simulink model "Demo_TrafficFlow_cs"  (Simulink 7.6)
settings: Solver=ode5  SolverMode=SingleTasking  StartTime=0.0  StopTime=60  FixedStep=1/20  RelTol=1e-3
PreLoadFcn:
  | check_NUTS_defined
  | try
  |     evalin('base', 'NUTS_defined;');
  | catch
  |     warningMsg = sprintf('PreScan Warning: \nUsing this model requires that MATLAB is configured for PreScan. The model may not display correctly.\n\n ==> Please open MATLAB with the dedicated buttons provided with PreScan');
  |     disp(warningMsg)
  |     warndlg(warningMsg,'WindowStyle','Modal');
  | end
  | 
  | model_closed = 0;
  | assignin('base',['model_closed_' bdroot],false);
InitFcn:
  | PreScanExperimentInitFcn(bdroot)
StopFcn:
  | PreScanExperimentStopFcn(bdroot)
undefined symbols (not defined in the file or in its folder): Advised_Speed_L1, Advised_Speed_L2, Advised_Speed_L3, DiscIntNeverNeededParam, spare_10, spare_5, spare_6, spare_7, spare_8, spare_9, State_L2, Throughput_L1, Throughput_L2, Throughput_L3, Velocity_L1, Velocity_L2, Velocity_L3
libraries: simulink x58, PreScanSimulinkLibrary x40, simulink_extras x32, PreScanUsefulBlocks x22, simulink_need_slupdate x3
(part 17 of 20)

== system Demo_TrafficFlow_cs/Toyota_Yaris_1/Simple_Longitudinal_ACC/LONGITUDINAL ACC & Dynamics ==
  note: DYNAMICS
  note: CONTROL
  note: CASE: DETECTION
      | vehicle in front detected
      | * postion control
      | * velocity control
  note: CASE: NO DETECTION
      | no vehicle in front detected
      | * velocity control
  Detected Range [m] : Inport
  Detected Velocity Vehicle [m/s] : Inport  Port=2
  Own Velocity Intention [km/h] : Inport  Port=3
  Safety Distance [m] : Inport  Port=4
  Gain1 : Gain  Gain=0.35
  Gain2 : Gain  Gain=1/3.6
  Rate Limiter2 : RateLimiter  RisingSlewLimit=3  FallingSlewLimit=-3  SampleTimeMode=inherited
  Sum : Sum  Inputs=-+|
  Sum2 : Sum  Inputs=++|
  Switch : Switch  Threshold=0.0001
  only forward driving : Saturate  UpperLimit=inf  LowerLimit=0
  Velocity [m/s] : Outport
  wires:
  Rate Limiter2/1 -> only forward driving/1
  only forward driving/1 -> Velocity [m/s]/1
  Sum/1 -> Gain1/1  "Control Error"
  Switch/1 -> Rate Limiter2/1
  Detected Range [m]/1 -> Switch/2, Sum/2
  Own Velocity Intention [km/h]/1 -> Gain2/1
  Gain2/1 -> Switch/3
  Sum2/1 -> Switch/1
  Detected Velocity Vehicle [m/s]/1 -> Sum2/2
  Safety Distance [m]/1 -> Sum/1
  Gain1/1 -> Sum2/1

== system Demo_TrafficFlow_cs/Toyota_Yaris_1/Trajectories/END OF TRACK REACHED & GO TO BUFFER ==
  Elapsed_Track [m] : Inport
  Length_of_Track [m] : Inport  Port=2
  Product1 : Product  RndMeth=Zero
  Relational Operator : RelationalOperator  OutDataTypeStr=boolean
  offset for displacement to  buffer of traffic LANE 3 : Constant  Tag=TrajSubSelector  Value=[0 27 0 0 0 0 0 0 0]
  Buffer_offset [m] : Outport
  End_of_Track_Reached [-] : Outport  Port=2
  wires:
  Length_of_Track [m]/1 -> Relational Operator/2
  Product1/1 -> Buffer_offset [m]/1
  Elapsed_Track [m]/1 -> Relational Operator/1
  Relational Operator/1 -> End_of_Track_Reached [-]/1, Product1/2
  offset for displacement to  buffer of traffic LANE 3/1 -> Product1/1

== system Demo_TrafficFlow_cs/Toyota_Yaris_1/Trajectories/ElapsedTrack1 ==
  Offset : Inport
  s : Inport  Port=2
  Mux : Mux  Inputs=3
  Mux1 : Mux  Inputs=3
  MuxState : Subsystem
  OffsetCor : Subsystem
  pitchAngle : Lookup  InputValues=s  Table=pitch  LookUpMeth=Interpolation-Use End Values
  rollAngle : Lookup  InputValues=s  Table=roll  LookUpMeth=Interpolation-Use End Values
  xpos : Lookup  InputValues=s  Table=x  LookUpMeth=Interpolation-Use End Values
  yawAngle : Lookup  InputValues=s  Table=yaw  LookUpMeth=Interpolation-Use End Values
  ypos : Lookup  InputValues=s  Table=y  LookUpMeth=Interpolation-Use End Values
  zpos : Lookup  InputValues=s  Table=z  LookUpMeth=Interpolation-Use End Values
  state : Outport
  wires:
  zpos/1 -> OffsetCor/3(z pos)
  rollAngle/1 -> OffsetCor/4(R angle)
  pitchAngle/1 -> OffsetCor/5(P angle)
  yawAngle/1 -> OffsetCor/6(Y angle)
  OffsetCor/6(Y angle_cor) -> Mux1/3
  OffsetCor/5(P angle_cor) -> Mux1/2
  OffsetCor/4(R angle_cor) -> Mux1/1
  OffsetCor/3(z pos_cor) -> Mux/3
  MuxState/1(State) -> state/1
  Mux/1 -> MuxState/1(xyzPos_[m])
  s/1 -> pitchAngle/1, yawAngle/1, rollAngle/1, zpos/1, ypos/1, xpos/1
  Offset/1 -> OffsetCor/7(Offset)
  xpos/1 -> OffsetCor/1(x pos)
  ypos/1 -> OffsetCor/2(y pos)
  OffsetCor/1(x pos_cor) -> Mux/1
  OffsetCor/2(y pos_cor) -> Mux/2
  Mux1/1 -> MuxState/2(RPYAngle_[rad])

== system Demo_TrafficFlow_cs/Toyota_Yaris_1/Trajectories/LANE CHANGE LEFT (cummulative y position;  multiple shift to right are possible) ==
  reset cum value : Inport
  velocity [m/s] : Inport  Port=2
  on/off [-] : Inport  Port=3
  Cumulative Lane Change : Subsystem
  Single LaneChange_Left : Subsystem
  Trigger : Subsystem  TreatAsAtomicUnit=on
  cum LC : Outport
  wires:
  Cumulative Lane Change/1(cum LC value) -> cum LC/1
  Single LaneChange_Left/2(single LC value) -> Cumulative Lane Change/3(single LC value)  "single LC value"
  reset cum value/1 -> Cumulative Lane Change/1(reset cum value)  "reset_signal"
  velocity [m/s]/1 -> Single LaneChange_Left/2(velocity [m/s])
  Trigger/1(Out1) -> Single LaneChange_Left/Enable
  Single LaneChange_Left/1(end LC reached) -> Cumulative Lane Change/2(end LC reached)  "end_reached"
  on/off [-]/1 -> Trigger/Trigger, Single LaneChange_Left/1(on/off [-])  "lane_change_signal"

== system Demo_TrafficFlow_cs/Toyota_Yaris_1/Trajectories/PathFollowerSva_7 ==
  From Workspace : FromWorkspace  VariableName=trajectory  SampleTime=TimeStep  OutputAfterFinalValue=Holding final value
  From Workspace1 : FromWorkspace  VariableName=trajectory  SampleTime=TimeStep  OutputAfterFinalValue=Setting to zero
  Mux : Mux  Inputs=2
  Selector1 : Selector  InputPortWidth=24  IndexOptions=Index vector (dialog)  Indices=[7:18]  OutputSizes=1
  Selector9 : Selector  InputPortWidth=24  IndexOptions=Index vector (dialog)  Indices=[1:6]  OutputSizes=1
  trajectory : Outport
  wires:
  Selector1/1 -> Mux/2
  Selector9/1 -> Mux/1
  Mux/1 -> trajectory/1
  From Workspace1/1 -> Selector1/1
  From Workspace/1 -> Selector9/1

== system Demo_TrafficFlow_cs/Toyota_Yaris_1/Trajectories/RESTART TRAJECT ==
  End_of_Track_Reached [-] : Inport
  ID_start_again [-] : Inport  Port=2
  objectID [-] : Inport  Port=3
  Logical Operator : Logic  AllPortsSameDT=off  OutDataTypeStr=boolean
  Memory : Memory
  Relational Operator1 : RelationalOperator  Operator===  OutDataTypeStr=boolean
  reset [-] : Outport
  Start_Traject [-] : Outport  Port=2
  wires:
  ID_start_again [-]/1 -> Relational Operator1/1
  Relational Operator1/1 -> reset [-]/1, Logical Operator/2
  Memory/1 -> Start_Traject [-]/1
  End_of_Track_Reached [-]/1 -> Logical Operator/1
  Logical Operator/1 -> Memory/1
  objectID [-]/1 -> Relational Operator1/2

== system Demo_TrafficFlow_cs/Toyota_Yaris_1/Trajectories/Random  LC generator ==
  Random Number : RandomNumber  Mean=0.5  Variance=0.1  SampleTime=0
  Rounding Function : Rounding  Operator=round
  0/1 : Outport
  wires:
  Rounding Function/1 -> 0/1/1
  Random Number/1 -> Rounding Function/1

== system Demo_TrafficFlow_cs/Toyota_Yaris_1/Trajectories/Traj2State1 ==
  Traj Org : Inport
  Offset : Inport  Port=2
  Add2 : Sum
  ApplyRotation : PreScanSimulinkLibrary/ApplyRotation  SystemSampleTime=-1
  ApplyRotation1 : PreScanSimulinkLibrary/ApplyRotation  SystemSampleTime=-1
  ApplyRotation2 : PreScanSimulinkLibrary/ApplyRotation  SystemSampleTime=-1
  Constant : Constant  Value=zeros(12,1)
  Mux : Mux  Inputs=6
  Mux1 : Mux  Inputs=2
  Mux{2,4..9} (7 alike) : Mux  Inputs=3
  Mux3 : Mux  Inputs=2
  Selector : Selector  InputPortWidth=6  IndexOptions=Index vector (dialog)  Indices=[4 5 6]  OutputSizes=1
  Selector1 : Selector  InputPortWidth=21  IndexOptions=Index vector (dialog)  Indices=[5]  OutputSizes=1
  Selector10 : Selector  InputPortWidth=21  IndexOptions=Index vector (dialog)  Indices=[2]  OutputSizes=1
  Selector11 : Selector  InputPortWidth=21  IndexOptions=Index vector (dialog)  Indices=[3]  OutputSizes=1
  Selector12 : Selector  InputPortWidth=21  IndexOptions=Index vector (dialog)  Indices=[1]  OutputSizes=1
  Selector13 : Selector  InputPortWidth=21  IndexOptions=Index vector (dialog)  Indices=[8]  OutputSizes=1
  Selector14 : Selector  InputPortWidth=21  IndexOptions=Index vector (dialog)  Indices=[9]  OutputSizes=1
  Selector15 : Selector  InputPortWidth=21  IndexOptions=Index vector (dialog)  Indices=[7]  OutputSizes=1
  Selector16 : Selector  InputPortWidth=21  IndexOptions=Index vector (dialog)  Indices=[14]  OutputSizes=1
  Selector17 : Selector  InputPortWidth=21  IndexOptions=Index vector (dialog)  Indices=[15]  OutputSizes=1
  Selector18 : Selector  InputPortWidth=21  IndexOptions=Index vector (dialog)  Indices=[13]  OutputSizes=1
  Selector19 : Selector  InputPortWidth=21  IndexOptions=Index vector (dialog)  Indices=[20]  OutputSizes=1
  Selector2 : Selector  InputPortWidth=21  IndexOptions=Index vector (dialog)  Indices=[6]  OutputSizes=1
  Selector20 : Selector  InputPortWidth=21  IndexOptions=Index vector (dialog)  Indices=[21]  OutputSizes=1
  Selector21 : Selector  InputPortWidth=21  IndexOptions=Index vector (dialog)  Indices=[19]  OutputSizes=1
  Selector3 : Selector  InputPortWidth=21  IndexOptions=Index vector (dialog)  Indices=[12]  OutputSizes=1
  Selector4 : Selector  InputPortWidth=21  IndexOptions=Index vector (dialog)  Indices=[17]  OutputSizes=1
  Selector5 : Selector  InputPortWidth=21  IndexOptions=Index vector (dialog)  Indices=[18]  OutputSizes=1
  Selector6 : Selector  InputPortWidth=21  IndexOptions=Index vector (dialog)  Indices=[16]  OutputSizes=1
  Selector7 : Selector  InputPortWidth=21  IndexOptions=Index vector (dialog)  Indices=[11]  OutputSizes=1
  Selector8 : Selector  InputPortWidth=21  IndexOptions=Index vector (dialog)  Indices=[10]  OutputSizes=1
  Selector9 : Selector  InputPortWidth=21  IndexOptions=Index vector (dialog)  Indices=[4]  OutputSizes=1
  Traj2State2 : Subsystem
  state : Outport
  wires:
  Mux9/1 -> ApplyRotation2/2, ApplyRotation1/2, ApplyRotation/2
  Selector20/1 -> Mux9/3
  Selector19/1 -> Mux9/2
  Selector21/1 -> Mux9/1
  Selector5/1 -> Mux8/3
  Selector4/1 -> Mux8/2
  Selector6/1 -> Mux8/1
  Mux8/1 -> Mux/6
  Mux6/1 -> ApplyRotation2/1
  Mux7/1 -> Mux/4
  ApplyRotation2/1 -> Mux/5
  Selector3/1 -> Mux7/3
  Selector7/1 -> Mux7/2
  Selector8/1 -> Mux7/1
  Mux4/1 -> Mux/2
  Mux5/1 -> ApplyRotation1/1
  ApplyRotation1/1 -> Mux/3
  Mux2/1 -> ApplyRotation/1
  ApplyRotation/1 -> Mux/1
  Selector2/1 -> Mux4/3
  Selector1/1 -> Mux4/2
  Selector9/1 -> Mux4/1
  Selector17/1 -> Mux6/3
  Selector16/1 -> Mux6/2
  Selector18/1 -> Mux6/1
  Selector14/1 -> Mux5/3
  Selector13/1 -> Mux5/2
  Selector15/1 -> Mux5/1
  Selector11/1 -> Mux2/3
  Selector10/1 -> Mux2/2
  Selector12/1 -> Mux2/1
  Mux3/1 -> Selector8/1, Selector7/1, Selector3/1, Selector18/1, Selector16/1, Selector17/1, Selector6/1, Selector20/1, Selector19/1, Selector21/1, Selector5/1, Selector4/1, Selector14/1, Selector13/1, Selector15/1, Selector2/1, Selector1/1, Selector9/1, Selector12/1, Selector10/1, Selector11/1
  Mux1/1 -> Add2/2
  Constant/1 -> Mux1/2
  Add2/1 -> Traj2State2/1(traj)
  Selector/1 -> Mux3/2
  Traj Org/1 -> Mux3/1
  Offset/1 -> Mux1/1, Selector/1
  Mux/1 -> Add2/1
  Traj2State2/1(state) -> state/1

== system Demo_TrafficFlow_cs/Tripod_1/REINSERT VEHICLE determine ID of Vehicle  which needs to start at beginning of /Enabled and Triggered Subsystem ==
  In1 : Inport
  Enable : EnablePort  PropagateVarSize=During execution
  Trigger : TriggerPort
  Out1 : Outport  OutputWhenDisabled=reset  InitialOutput=[0]
  wires:
  In1/1 -> Out1/1

== system Demo_TrafficFlow_cs/Tripod_1/RFBeacon_4_Rx_Demux/Reorder ==
  In : Inport
  Selector1 : Selector  InputPortWidth=InputPortWidth  IndexOptions=Index vector (dialog)  Indices=Elements  OutputSizes=1
  Out : Outport
  wires:
  Selector1/1 -> Out/1
  In/1 -> Selector1/1

== system Demo_TrafficFlow_cs/Tripod_1/RFBeacon_4_Rx_Demux/compas converter ==
  rotz [rad] : Inport
  cart2compas : Subsystem
  rad2deg : Gain  Gain=180/pi
  heading [deg] : Outport
  wires:
  rad2deg/1 -> cart2compas/1(Angle [deg])
  rotz [rad]/1 -> rad2deg/1
  cart2compas/1(heading [deg]) -> heading [deg]/1

== system Demo_TrafficFlow_cs/Tripod_2/REINSERT VEHICLE determine ID of Vehicle  which needs to start at beginning of /Enabled and Triggered Subsystem ==
  In1 : Inport
  Enable : EnablePort  PropagateVarSize=During execution
  Trigger : TriggerPort
  Out1 : Outport  OutputWhenDisabled=reset  InitialOutput=[0]
  wires:
  In1/1 -> Out1/1

== system Demo_TrafficFlow_cs/Tripod_2/RFBeacon_5_Rx_Demux/Reorder ==
  In : Inport
  Selector1 : Selector  InputPortWidth=InputPortWidth  IndexOptions=Index vector (dialog)  Indices=Elements  OutputSizes=1
  Out : Outport
  wires:
  Selector1/1 -> Out/1
  In/1 -> Selector1/1

== system Demo_TrafficFlow_cs/Tripod_2/RFBeacon_5_Rx_Demux/compas converter ==
  rotz [rad] : Inport
  cart2compas : Subsystem
  rad2deg : Gain  Gain=180/pi
  heading [deg] : Outport
  wires:
  rad2deg/1 -> cart2compas/1(Angle [deg])
  rotz [rad]/1 -> rad2deg/1
  cart2compas/1(heading [deg]) -> heading [deg]/1

== system Demo_TrafficFlow_cs/Audi_A8_1/SELF_Demux/compas converter/cart2compas ==
  Angle [deg] : Inport
  Constant : Constant  Value=90
  Constant1 : Constant  Value=360
  Gain : Gain  Gain=1/360
  Gain1 : Gain  Gain=360
  Gain2 : Gain  Gain=-1
  Rounding Function : Rounding
  Sum : Sum  Inputs=|-+
  Sum1 : Sum  Inputs=-+|
  Sum2 : Sum  Inputs=++|
  heading [deg] : Outport
  wires:
  Sum2/1 -> heading [deg]/1
  Constant1/1 -> Sum2/1
  Gain2/1 -> Sum2/2
  Constant/1 -> Sum1/1
  Sum1/1 -> Sum/2, Gain/1
  Sum/1 -> Gain2/1
  Angle [deg]/1 -> Sum1/2
  Gain1/1 -> Sum/1
  Rounding Function/1 -> Gain1/1
  Gain/1 -> Rounding Function/1

== system Demo_TrafficFlow_cs/Audi_A8_1/Trajectories/ElapsedTrack1/MuxState ==
  xyzPos_[m] : Inport
  RPYAngle_[rad] : Inport  Port=2
  Demux : Demux  Outputs=3
  Discrete Derivative : simulink/Discrete/Discrete Derivative  SystemSampleTime=-1  gainval=1.0  ICPrevScaledInput=0.0  OutMin=[]  OutMax=[]  OutDataType=fixdt(1, 16)  OutScaling=2^-10  DoSatur=off
  Mux : Mux  Inputs=3
  Mux1 : Mux  Inputs=3
  Substitute 90 & 270 deg (and its plurals) : Subsystem
  State : Outport
  wires:
  Mux1/1 -> Mux/3
  Discrete Derivative/1 -> Mux/2
  RPYAngle_[rad]/1 -> Demux/1
  Demux/3 -> Mux1/3
  Demux/2 -> Substitute 90 & 270 deg (and its plurals)/1(Angle [rad])
  Demux/1 -> Mux1/1
  xyzPos_[m]/1 -> Discrete Derivative/1, Mux/1
  Mux/1 -> State/1
  Substitute 90 & 270 deg (and its plurals)/1(Subst Angle [rad]) -> Mux1/2

== system Demo_TrafficFlow_cs/Audi_A8_1/Trajectories/ElapsedTrack1/OffsetCor ==
  x pos : Inport
  y pos : Inport  Port=2
  z pos : Inport  Port=3
  R angle : Inport  Port=4
  P angle : Inport  Port=5
  Y angle : Inport  Port=6
  Offset : Inport  Port=7
  ApplyRotation : PreScanSimulinkLibrary/ApplyRotation  SystemSampleTime=-1
  Demux : Demux  Outputs=6
  Mux : Mux  Inputs=2
  Mux1 : Mux  Inputs=3
  Mux2 : Mux  Inputs=3
  Mux3 : Mux  Inputs=3
  Selector2 : Selector  InputPortWidth=6  IndexOptions=Index vector (dialog)  Indices=[4]  OutputSizes=1
  Selector3 : Selector  InputPortWidth=6  IndexOptions=Index vector (dialog)  Indices=[5]  OutputSizes=1
  Selector4 : Selector  InputPortWidth=6  IndexOptions=Index vector (dialog)  Indices=[6]  OutputSizes=1
  Sum : Sum  Inputs=|++
  x pos_cor : Outport
  y pos_cor : Outport  Port=2
  z pos_cor : Outport  Port=3
  R angle_cor : Outport  Port=4
  P angle_cor : Outport  Port=5
  Y angle_cor : Outport  Port=6
  wires:
  Mux3/1 -> ApplyRotation/1
  ApplyRotation/1 -> Mux/1
  Mux2/1 -> ApplyRotation/2
  Selector4/1 -> Mux2/3
  Selector3/1 -> Mux2/2
  Selector2/1 -> Mux2/1
  Mux1/1 -> Mux/2
  Y angle/1 -> Mux1/3
  P angle/1 -> Mux1/2
  R angle/1 -> Mux1/1
  Demux/1 -> x pos_cor/1
  Demux/2 -> y pos_cor/1
  Demux/3 -> z pos_cor/1
  Demux/4 -> R angle_cor/1
  Demux/5 -> P angle_cor/1
  Demux/6 -> Y angle_cor/1
  z pos/1 -> Mux3/3
  y pos/1 -> Mux3/2
  x pos/1 -> Mux3/1
  Offset/1 -> Selector4/1, Selector3/1, Selector2/1, Sum/2
  Mux/1 -> Sum/1
  Sum/1 -> Demux/1

== system Demo_TrafficFlow_cs/Audi_A8_1/Trajectories/LANE CHANGE LEFT (cummulative y position;  multiple shift to left are possible)/Cumulative Lane Change ==
  reset cum value : Inport
  end LC reached : Inport  Port=2
  single LC value : Inport  Port=3
  Logical Operator1 : Logic  Operator=NOT
  Memory1 : Memory
  Memory2 : Memory
  Sum : Sum  Inputs=|++
  Triggered Subsystem : Subsystem  TreatAsAtomicUnit=on
  cum LC value : Outport  InitialOutput=0
  wires:
  Sum/1 -> cum LC value/1  "value"
  end LC reached/1 -> Triggered Subsystem/Trigger
  Triggered Subsystem/1(Out1) -> Memory2/1, Sum/1  "hold_value"
  Memory1/1 -> Triggered Subsystem/1(In1)
  Memory2/1 -> Triggered Subsystem/2(In2)
  single LC value/1 -> Sum/2, Memory1/1
  reset cum value/1 -> Logical Operator1/1
  Logical Operator1/1 -> Triggered Subsystem/Enable

== system Demo_TrafficFlow_cs/Audi_A8_1/Trajectories/LANE CHANGE LEFT (cummulative y position;  multiple shift to left are possible)/Single LaneChange_Left ==
  on/off [-] : Inport
  velocity [m/s] : Inport  Port=2
  Enable : EnablePort  PropagateVarSize=During execution
  ElapsedTrack2 : Subsystem  mask(PreScan Demux Block:){Filename='Path_LC_Left.mat'}
      mask prompts: Filename "Name path-file"
      mask init:
      | %route=load(['trajectories/',Filename]);
      | route=load(Filename);
      | s=route.path(1,:);
      | x=route.path(2,:);
      | y=route.path(3,:);
      | yaw=route.path(4,:);
  Gain : Gain  Gain="[0 1 0   0 1 0   0 0 1]"
  Integrator2 : Integrator  ExternalReset=rising  UpperSaturationLimit=100  LowerSaturationLimit=-1e-3
  Logical Operator1 : Logic  Operator=NOT  AllPortsSameDT=off  OutDataTypeStr=boolean
  Product1 : Product  RndMeth=Zero
  Relational Operator : RelationalOperator  Operator=<  OutDataTypeStr=boolean
  Trajectory_4_Offset1 : Constant  Value=[0 0 0]
  ini_cond3 : Constant  Value=s(end)
  end LC reached : Outport  InitialOutput=1
  single LC value : Outport  Port=2  InitialOutput=0
  wires:
  on/off [-]/1 -> Integrator2/2  "trigger"
  Relational Operator/1 -> Product1/2, Logical Operator1/1  "end_NOT_reached"
  ini_cond3/1 -> Relational Operator/2
  Gain/1 -> Product1/1
  Integrator2/1 -> Relational Operator/1, ElapsedTrack2/2(s)
  Trajectory_4_Offset1/1 -> ElapsedTrack2/1(Offset)
  ElapsedTrack2/1(state) -> Gain/1
  Product1/1 -> single LC value/1
  velocity [m/s]/1 -> Integrator2/1
  Logical Operator1/1 -> end LC reached/1  "end_reached"

== system Demo_TrafficFlow_cs/Audi_A8_1/Trajectories/LANE CHANGE LEFT (cummulative y position;  multiple shift to left are possible)/Trigger ==
  Trigger : TriggerPort
  ini_cond10 : Constant
  Out1 : Outport  InitialOutput=[0]
  wires:
  ini_cond10/1 -> Out1/1

== system Demo_TrafficFlow_cs/Audi_A8_1/Trajectories/Traj2State1/Traj2State2 ==
  traj : Inport
  MuxState : Subsystem
  Traj2MuxState_Ext : Subsystem
  state : Outport
  wires:
  Traj2MuxState_Ext/9(Y [rad]) -> MuxState/9(Y [rad])
  Traj2MuxState_Ext/8(P [rad]) -> MuxState/8(P [rad])
  Traj2MuxState_Ext/7(R [rad]) -> MuxState/7(R [rad])
  Traj2MuxState_Ext/6(dz [m/s]) -> MuxState/6(dz [m/s])
  Traj2MuxState_Ext/5(dy [m/s]) -> MuxState/5(dy [m/s])
  Traj2MuxState_Ext/4(dx [m/s]) -> MuxState/4(dx [m/s])
  Traj2MuxState_Ext/3(z [m]) -> MuxState/3(z [m])
  Traj2MuxState_Ext/2(y [m]) -> MuxState/2(y [m])
  Traj2MuxState_Ext/1(x [m]) -> MuxState/1(x [m])
  MuxState/1(state) -> state/1
  traj/1 -> Traj2MuxState_Ext/1(Traj)

== system Demo_TrafficFlow_cs/BMW_X5_1/SELF_Demux/compas converter/cart2compas ==
  Angle [deg] : Inport
  Constant : Constant  Value=90
  Constant1 : Constant  Value=360
  Gain : Gain  Gain=1/360
  Gain1 : Gain  Gain=360
  Gain2 : Gain  Gain=-1
  Rounding Function : Rounding
  Sum : Sum  Inputs=|-+
  Sum1 : Sum  Inputs=-+|
  Sum2 : Sum  Inputs=++|
  heading [deg] : Outport
  wires:
  Sum2/1 -> heading [deg]/1
  Constant1/1 -> Sum2/1
  Gain2/1 -> Sum2/2
  Constant/1 -> Sum1/1
  Sum1/1 -> Sum/2, Gain/1
  Sum/1 -> Gain2/1
  Angle [deg]/1 -> Sum1/2
  Gain1/1 -> Sum/1
  Rounding Function/1 -> Gain1/1
  Gain/1 -> Rounding Function/1

== system Demo_TrafficFlow_cs/BMW_X5_1/Trajectories/ElapsedTrack1/MuxState ==
  xyzPos_[m] : Inport
  RPYAngle_[rad] : Inport  Port=2
  Demux : Demux  Outputs=3
  Discrete Derivative : simulink/Discrete/Discrete Derivative  SystemSampleTime=-1  gainval=1.0  ICPrevScaledInput=0.0  OutMin=[]  OutMax=[]  OutDataType=fixdt(1, 16)  OutScaling=2^-10  DoSatur=off
  Mux : Mux  Inputs=3
  Mux1 : Mux  Inputs=3
  Substitute 90 & 270 deg (and its plurals) : Subsystem
  State : Outport
  wires:
  Mux1/1 -> Mux/3
  Discrete Derivative/1 -> Mux/2
  RPYAngle_[rad]/1 -> Demux/1
  Demux/3 -> Mux1/3
  Demux/2 -> Substitute 90 & 270 deg (and its plurals)/1(Angle [rad])
  Demux/1 -> Mux1/1
  xyzPos_[m]/1 -> Discrete Derivative/1, Mux/1
  Mux/1 -> State/1
  Substitute 90 & 270 deg (and its plurals)/1(Subst Angle [rad]) -> Mux1/2

== system Demo_TrafficFlow_cs/BMW_X5_1/Trajectories/ElapsedTrack1/OffsetCor ==
  x pos : Inport
  y pos : Inport  Port=2
  z pos : Inport  Port=3
  R angle : Inport  Port=4
  P angle : Inport  Port=5
  Y angle : Inport  Port=6
  Offset : Inport  Port=7
  ApplyRotation : PreScanSimulinkLibrary/ApplyRotation  SystemSampleTime=-1
  Demux : Demux  Outputs=6
  Mux : Mux  Inputs=2
  Mux1 : Mux  Inputs=3
  Mux2 : Mux  Inputs=3
  Mux3 : Mux  Inputs=3
  Selector2 : Selector  InputPortWidth=6  IndexOptions=Index vector (dialog)  Indices=[4]  OutputSizes=1
  Selector3 : Selector  InputPortWidth=6  IndexOptions=Index vector (dialog)  Indices=[5]  OutputSizes=1
  Selector4 : Selector  InputPortWidth=6  IndexOptions=Index vector (dialog)  Indices=[6]  OutputSizes=1
  Sum : Sum  Inputs=|++
  x pos_cor : Outport
  y pos_cor : Outport  Port=2
  z pos_cor : Outport  Port=3
  R angle_cor : Outport  Port=4
  P angle_cor : Outport  Port=5
  Y angle_cor : Outport  Port=6
  wires:
  Mux3/1 -> ApplyRotation/1
  ApplyRotation/1 -> Mux/1
  Mux2/1 -> ApplyRotation/2
  Selector4/1 -> Mux2/3
  Selector3/1 -> Mux2/2
  Selector2/1 -> Mux2/1
  Mux1/1 -> Mux/2
  Y angle/1 -> Mux1/3
  P angle/1 -> Mux1/2
  R angle/1 -> Mux1/1
  Demux/1 -> x pos_cor/1
  Demux/2 -> y pos_cor/1
  Demux/3 -> z pos_cor/1
  Demux/4 -> R angle_cor/1
  Demux/5 -> P angle_cor/1
  Demux/6 -> Y angle_cor/1
  z pos/1 -> Mux3/3
  y pos/1 -> Mux3/2
  x pos/1 -> Mux3/1
  Offset/1 -> Selector4/1, Selector3/1, Selector2/1, Sum/2
  Mux/1 -> Sum/1
  Sum/1 -> Demux/1

== system Demo_TrafficFlow_cs/BMW_X5_1/Trajectories/LANE CHANGE LEFT (cummulative y position;  multiple shift to right are possible)/Cumulative Lane Change ==
  reset cum value : Inport
  end LC reached : Inport  Port=2
  single LC value : Inport  Port=3
  Logical Operator1 : Logic  Operator=NOT
  Memory1 : Memory
  Memory2 : Memory
  Sum : Sum  Inputs=|++
  Triggered Subsystem : Subsystem  TreatAsAtomicUnit=on
  cum LC value : Outport  InitialOutput=0
  wires:
  Sum/1 -> cum LC value/1  "value"
  end LC reached/1 -> Triggered Subsystem/Trigger
  Triggered Subsystem/1(Out1) -> Memory2/1, Sum/1  "hold_value"
  Memory1/1 -> Triggered Subsystem/1(In1)
  Memory2/1 -> Triggered Subsystem/2(In2)
  single LC value/1 -> Sum/2, Memory1/1
  reset cum value/1 -> Logical Operator1/1
  Logical Operator1/1 -> Triggered Subsystem/Enable

== system Demo_TrafficFlow_cs/BMW_X5_1/Trajectories/LANE CHANGE LEFT (cummulative y position;  multiple shift to right are possible)/Single LaneChange_Left ==
  on/off [-] : Inport
  velocity [m/s] : Inport  Port=2
  Enable : EnablePort  PropagateVarSize=During execution
  ElapsedTrack2 : Subsystem  mask(PreScan Demux Block:){Filename='Path_LC_Left.mat'}
      mask prompts: Filename "Name path-file"
      mask init:
      | %route=load(['trajectories/',Filename]);
      | route=load(Filename);
      | s=route.path(1,:);
      | x=route.path(2,:);
      | y=route.path(3,:);
      | yaw=route.path(4,:);
  Gain : Gain  Gain="[0 1 0   0 1 0   0 0 1]"
  Integrator2 : Integrator  ExternalReset=rising  UpperSaturationLimit=100  LowerSaturationLimit=-1e-3
  Logical Operator1 : Logic  Operator=NOT  AllPortsSameDT=off  OutDataTypeStr=boolean
  Product1 : Product  RndMeth=Zero
  Relational Operator : RelationalOperator  Operator=<  OutDataTypeStr=boolean
  Trajectory_4_Offset1 : Constant  Value=[0 0 0]
  ini_cond3 : Constant  Value=s(end)
  end LC reached : Outport  InitialOutput=1
  single LC value : Outport  Port=2  InitialOutput=0
  wires:
  on/off [-]/1 -> Integrator2/2  "trigger"
  Relational Operator/1 -> Product1/2, Logical Operator1/1  "end_NOT_reached"
  ini_cond3/1 -> Relational Operator/2
  Gain/1 -> Product1/1
  Integrator2/1 -> Relational Operator/1, ElapsedTrack2/2(s)
  Trajectory_4_Offset1/1 -> ElapsedTrack2/1(Offset)
  ElapsedTrack2/1(state) -> Gain/1
  Product1/1 -> single LC value/1
  velocity [m/s]/1 -> Integrator2/1
  Logical Operator1/1 -> end LC reached/1  "end_reached"

== system Demo_TrafficFlow_cs/BMW_X5_1/Trajectories/LANE CHANGE LEFT (cummulative y position;  multiple shift to right are possible)/Trigger ==
  Trigger : TriggerPort
  ini_cond10 : Constant
  Out1 : Outport  InitialOutput=[0]
  wires:
  ini_cond10/1 -> Out1/1

== system Demo_TrafficFlow_cs/BMW_X5_1/Trajectories/Traj2State1/Traj2State2 ==
  traj : Inport
  MuxState : Subsystem
  Traj2MuxState_Ext : Subsystem
  state : Outport
  wires:
  Traj2MuxState_Ext/9(Y [rad]) -> MuxState/9(Y [rad])
  Traj2MuxState_Ext/8(P [rad]) -> MuxState/8(P [rad])
  Traj2MuxState_Ext/7(R [rad]) -> MuxState/7(R [rad])
  Traj2MuxState_Ext/6(dz [m/s]) -> MuxState/6(dz [m/s])
  Traj2MuxState_Ext/5(dy [m/s]) -> MuxState/5(dy [m/s])
  Traj2MuxState_Ext/4(dx [m/s]) -> MuxState/4(dx [m/s])
  Traj2MuxState_Ext/3(z [m]) -> MuxState/3(z [m])
  Traj2MuxState_Ext/2(y [m]) -> MuxState/2(y [m])
  Traj2MuxState_Ext/1(x [m]) -> MuxState/1(x [m])
  MuxState/1(state) -> state/1
  traj/1 -> Traj2MuxState_Ext/1(Traj)

== system Demo_TrafficFlow_cs/Citroen_C3_1/SELF_Demux/compas converter/cart2compas ==
  Angle [deg] : Inport
  Constant : Constant  Value=90
  Constant1 : Constant  Value=360
  Gain : Gain  Gain=1/360
  Gain1 : Gain  Gain=360
  Gain2 : Gain  Gain=-1
  Rounding Function : Rounding
  Sum : Sum  Inputs=|-+
  Sum1 : Sum  Inputs=-+|
  Sum2 : Sum  Inputs=++|
  heading [deg] : Outport
  wires:
  Sum2/1 -> heading [deg]/1
  Constant1/1 -> Sum2/1
  Gain2/1 -> Sum2/2
  Constant/1 -> Sum1/1
  Sum1/1 -> Sum/2, Gain/1
  Sum/1 -> Gain2/1
  Angle [deg]/1 -> Sum1/2
  Gain1/1 -> Sum/1
  Rounding Function/1 -> Gain1/1
  Gain/1 -> Rounding Function/1

== system Demo_TrafficFlow_cs/Citroen_C3_1/Trajectories/ElapsedTrack1/MuxState ==
  xyzPos_[m] : Inport
  RPYAngle_[rad] : Inport  Port=2
  Demux : Demux  Outputs=3
  Discrete Derivative : simulink/Discrete/Discrete Derivative  SystemSampleTime=-1  gainval=1.0  ICPrevScaledInput=0.0  OutMin=[]  OutMax=[]  OutDataType=fixdt(1, 16)  OutScaling=2^-10  DoSatur=off
  Mux : Mux  Inputs=3
  Mux1 : Mux  Inputs=3
  Substitute 90 & 270 deg (and its plurals) : Subsystem
  State : Outport
  wires:
  Mux1/1 -> Mux/3
  Discrete Derivative/1 -> Mux/2
  RPYAngle_[rad]/1 -> Demux/1
  Demux/3 -> Mux1/3
  Demux/2 -> Substitute 90 & 270 deg (and its plurals)/1(Angle [rad])
  Demux/1 -> Mux1/1
  xyzPos_[m]/1 -> Discrete Derivative/1, Mux/1
  Mux/1 -> State/1
  Substitute 90 & 270 deg (and its plurals)/1(Subst Angle [rad]) -> Mux1/2

== system Demo_TrafficFlow_cs/Citroen_C3_1/Trajectories/ElapsedTrack1/OffsetCor ==
  x pos : Inport
  y pos : Inport  Port=2
  z pos : Inport  Port=3
  R angle : Inport  Port=4
  P angle : Inport  Port=5
  Y angle : Inport  Port=6
  Offset : Inport  Port=7
  ApplyRotation : PreScanSimulinkLibrary/ApplyRotation  SystemSampleTime=-1
  Demux : Demux  Outputs=6
  Mux : Mux  Inputs=2
  Mux1 : Mux  Inputs=3
  Mux2 : Mux  Inputs=3
  Mux3 : Mux  Inputs=3
  Selector2 : Selector  InputPortWidth=6  IndexOptions=Index vector (dialog)  Indices=[4]  OutputSizes=1
  Selector3 : Selector  InputPortWidth=6  IndexOptions=Index vector (dialog)  Indices=[5]  OutputSizes=1
  Selector4 : Selector  InputPortWidth=6  IndexOptions=Index vector (dialog)  Indices=[6]  OutputSizes=1
  Sum : Sum  Inputs=|++
  x pos_cor : Outport
  y pos_cor : Outport  Port=2
  z pos_cor : Outport  Port=3
  R angle_cor : Outport  Port=4
  P angle_cor : Outport  Port=5
  Y angle_cor : Outport  Port=6
  wires:
  Mux3/1 -> ApplyRotation/1
  ApplyRotation/1 -> Mux/1
  Mux2/1 -> ApplyRotation/2
  Selector4/1 -> Mux2/3
  Selector3/1 -> Mux2/2
  Selector2/1 -> Mux2/1
  Mux1/1 -> Mux/2
  Y angle/1 -> Mux1/3
  P angle/1 -> Mux1/2
  R angle/1 -> Mux1/1
  Demux/1 -> x pos_cor/1
  Demux/2 -> y pos_cor/1
  Demux/3 -> z pos_cor/1
  Demux/4 -> R angle_cor/1
  Demux/5 -> P angle_cor/1
  Demux/6 -> Y angle_cor/1
  z pos/1 -> Mux3/3
  y pos/1 -> Mux3/2
  x pos/1 -> Mux3/1
  Offset/1 -> Selector4/1, Selector3/1, Selector2/1, Sum/2
  Mux/1 -> Sum/1
  Sum/1 -> Demux/1

== system Demo_TrafficFlow_cs/Citroen_C3_1/Trajectories/LANE CHANGE LEFT (cummulative y position;  multiple shift to left are possible)/Cumulative Lane Change ==
  reset cum value : Inport
  end LC reached : Inport  Port=2
  single LC value : Inport  Port=3
  Logical Operator1 : Logic  Operator=NOT
  Memory1 : Memory
  Memory2 : Memory
  Sum : Sum  Inputs=|++
  Triggered Subsystem : Subsystem  TreatAsAtomicUnit=on
  cum LC value : Outport  InitialOutput=0
  wires:
  Sum/1 -> cum LC value/1  "value"
  end LC reached/1 -> Triggered Subsystem/Trigger
  Triggered Subsystem/1(Out1) -> Memory2/1, Sum/1  "hold_value"
  Memory1/1 -> Triggered Subsystem/1(In1)
  Memory2/1 -> Triggered Subsystem/2(In2)
  single LC value/1 -> Sum/2, Memory1/1
  reset cum value/1 -> Logical Operator1/1
  Logical Operator1/1 -> Triggered Subsystem/Enable

== system Demo_TrafficFlow_cs/Citroen_C3_1/Trajectories/LANE CHANGE LEFT (cummulative y position;  multiple shift to left are possible)/Single LaneChange_Left ==
  on/off [-] : Inport
  velocity [m/s] : Inport  Port=2
  Enable : EnablePort  PropagateVarSize=During execution
  ElapsedTrack2 : Subsystem  mask(PreScan Demux Block:){Filename='Path_LC_Left.mat'}
      mask prompts: Filename "Name path-file"
      mask init:
      | %route=load(['trajectories/',Filename]);
      | route=load(Filename);
      | s=route.path(1,:);
      | x=route.path(2,:);
      | y=route.path(3,:);
      | yaw=route.path(4,:);
  Gain : Gain  Gain="[0 1 0   0 1 0   0 0 1]"
  Integrator2 : Integrator  ExternalReset=rising  UpperSaturationLimit=100  LowerSaturationLimit=-1e-3
  Logical Operator1 : Logic  Operator=NOT  AllPortsSameDT=off  OutDataTypeStr=boolean
  Product1 : Product  RndMeth=Zero
  Relational Operator : RelationalOperator  Operator=<  OutDataTypeStr=boolean
  Trajectory_4_Offset1 : Constant  Value=[0 0 0]
  ini_cond3 : Constant  Value=s(end)
  end LC reached : Outport  InitialOutput=1
  single LC value : Outport  Port=2  InitialOutput=0
  wires:
  on/off [-]/1 -> Integrator2/2  "trigger"
  Relational Operator/1 -> Product1/2, Logical Operator1/1  "end_NOT_reached"
  ini_cond3/1 -> Relational Operator/2
  Gain/1 -> Product1/1
  Integrator2/1 -> Relational Operator/1, ElapsedTrack2/2(s)
  Trajectory_4_Offset1/1 -> ElapsedTrack2/1(Offset)
  ElapsedTrack2/1(state) -> Gain/1
  Product1/1 -> single LC value/1
  velocity [m/s]/1 -> Integrator2/1
  Logical Operator1/1 -> end LC reached/1  "end_reached"

== system Demo_TrafficFlow_cs/Citroen_C3_1/Trajectories/LANE CHANGE LEFT (cummulative y position;  multiple shift to left are possible)/Trigger ==
  Trigger : TriggerPort
  ini_cond10 : Constant
  Out1 : Outport  InitialOutput=[0]
  wires:
  ini_cond10/1 -> Out1/1

== system Demo_TrafficFlow_cs/Citroen_C3_1/Trajectories/Traj2State1/Traj2State2 ==
  traj : Inport
  MuxState : Subsystem
  Traj2MuxState_Ext : Subsystem
  state : Outport
  wires:
  Traj2MuxState_Ext/9(Y [rad]) -> MuxState/9(Y [rad])
  Traj2MuxState_Ext/8(P [rad]) -> MuxState/8(P [rad])
  Traj2MuxState_Ext/7(R [rad]) -> MuxState/7(R [rad])
  Traj2MuxState_Ext/6(dz [m/s]) -> MuxState/6(dz [m/s])
  Traj2MuxState_Ext/5(dy [m/s]) -> MuxState/5(dy [m/s])
  Traj2MuxState_Ext/4(dx [m/s]) -> MuxState/4(dx [m/s])
  Traj2MuxState_Ext/3(z [m]) -> MuxState/3(z [m])
  Traj2MuxState_Ext/2(y [m]) -> MuxState/2(y [m])
  Traj2MuxState_Ext/1(x [m]) -> MuxState/1(x [m])
  MuxState/1(state) -> state/1
  traj/1 -> Traj2MuxState_Ext/1(Traj)

== system Demo_TrafficFlow_cs/Control_Unit/Antenna_Rx_7_Demux/compas converter/cart2compas ==
  Angle [deg] : Inport
  Constant : Constant  Value=90
  Constant1 : Constant  Value=360
  Gain : Gain  Gain=1/360
  Gain1 : Gain  Gain=360
  Gain2 : Gain  Gain=-1
  Rounding Function : Rounding
  Sum : Sum  Inputs=|-+
  Sum1 : Sum  Inputs=-+|
  Sum2 : Sum  Inputs=++|
  heading [deg] : Outport
  wires:
  Gain/1 -> Rounding Function/1
  Rounding Function/1 -> Gain1/1
  Gain1/1 -> Sum/1
  Angle [deg]/1 -> Sum1/2
  Sum/1 -> Gain2/1
  Sum1/1 -> Gain/1, Sum/2
  Constant/1 -> Sum1/1
  Gain2/1 -> Sum2/2
  Constant1/1 -> Sum2/1
  Sum2/1 -> heading [deg]/1

== system Demo_TrafficFlow_cs/Control_Unit/Antenna_Rx_8_Demux/compas converter/cart2compas ==
  Angle [deg] : Inport
  Constant : Constant  Value=90
  Constant1 : Constant  Value=360
  Gain : Gain  Gain=1/360
  Gain1 : Gain  Gain=360
  Gain2 : Gain  Gain=-1
  Rounding Function : Rounding
  Sum : Sum  Inputs=|-+
  Sum1 : Sum  Inputs=-+|
  Sum2 : Sum  Inputs=++|
  heading [deg] : Outport
  wires:
  Gain/1 -> Rounding Function/1
  Rounding Function/1 -> Gain1/1
  Gain1/1 -> Sum/1
  Angle [deg]/1 -> Sum1/2
  Sum/1 -> Gain2/1
  Sum1/1 -> Gain/1, Sum/2
  Constant/1 -> Sum1/1
  Gain2/1 -> Sum2/2
  Constant1/1 -> Sum2/1
  Sum2/1 -> heading [deg]/1

== system Demo_TrafficFlow_cs/Control_Unit/Antenna_Rx_9_Demux/compas converter/cart2compas ==
  Angle [deg] : Inport
  Constant : Constant  Value=90
  Constant1 : Constant  Value=360
  Gain : Gain  Gain=1/360
  Gain1 : Gain  Gain=360
  Gain2 : Gain  Gain=-1
  Rounding Function : Rounding
  Sum : Sum  Inputs=|-+
  Sum1 : Sum  Inputs=-+|
  Sum2 : Sum  Inputs=++|
  heading [deg] : Outport
  wires:
  Gain/1 -> Rounding Function/1
  Rounding Function/1 -> Gain1/1
  Gain1/1 -> Sum/1
  Angle [deg]/1 -> Sum1/2
  Sum/1 -> Gain2/1
  Sum1/1 -> Gain/1, Sum/2
  Constant/1 -> Sum1/1
  Gain2/1 -> Sum2/2
  Constant1/1 -> Sum2/1
  Sum2/1 -> heading [deg]/1

== system Demo_TrafficFlow_cs/Control_Unit/Control Lane2/Lane2 open for Lane1/Counter ==
  note: This counter only works in case of a fixed time step solver
  Sum : Sum  Inputs=|++  SaturateOnIntegerOverflow=on  InputSameDT=on  OutDataTypeStr=Inherit: Same as first input
  Sum1 : Sum  Inputs=|+-  SaturateOnIntegerOverflow=on  InputSameDT=on  OutDataTypeStr=Inherit: Same as first input
  Unit Delay : UnitDelay  SampleTime=-1
  constant1 : Constant  Value=eval(get_param(bdroot,'FixedStep'))
  Out1 : Outport
  wires:
  Sum1/1 -> Out1/1
  Unit Delay/1 -> Sum/2
  constant1/1 -> Sum/1, Sum1/2
  Sum/1 -> Sum1/1, Unit Delay/1

== system Demo_TrafficFlow_cs/Control_Unit/Control Lane2/Lane2 open for Lane3/Counter ==
  note: This counter only works in case of a fixed time step solver
  Sum : Sum  Inputs=|++  SaturateOnIntegerOverflow=on  InputSameDT=on  OutDataTypeStr=Inherit: Same as first input
  Sum1 : Sum  Inputs=|+-  SaturateOnIntegerOverflow=on  InputSameDT=on  OutDataTypeStr=Inherit: Same as first input
  Unit Delay : UnitDelay  SampleTime=-1
  constant1 : Constant  Value=eval(get_param(bdroot,'FixedStep'))
  Out1 : Outport
  wires:
  Sum1/1 -> Out1/1
  Unit Delay/1 -> Sum/2
  constant1/1 -> Sum/1, Sum1/2
  Sum/1 -> Sum1/1, Unit Delay/1

== system Demo_TrafficFlow_cs/HighwayOverhang_1/Antenna_Rx_2_Demux/compas converter/cart2compas ==
  Angle [deg] : Inport
  Constant : Constant  Value=90
  Constant1 : Constant  Value=360
  Gain : Gain  Gain=1/360
  Gain1 : Gain  Gain=360
  Gain2 : Gain  Gain=-1
  Rounding Function : Rounding
  Sum : Sum  Inputs=|-+
  Sum1 : Sum  Inputs=-+|
  Sum2 : Sum  Inputs=++|
  heading [deg] : Outport
  wires:
  Gain/1 -> Rounding Function/1
  Rounding Function/1 -> Gain1/1
  Gain1/1 -> Sum/1
  Angle [deg]/1 -> Sum1/2
  Sum/1 -> Gain2/1
  Sum1/1 -> Gain/1, Sum/2
  Constant/1 -> Sum1/1
  Gain2/1 -> Sum2/2
  Constant1/1 -> Sum2/1
  Sum2/1 -> heading [deg]/1

== system Demo_TrafficFlow_cs/HighwayOverhang_1/RFBeacon_1_Rx_Demux/compas converter/cart2compas ==
  Angle [deg] : Inport
  Constant : Constant  Value=90
  Constant1 : Constant  Value=360
  Gain : Gain  Gain=1/360
  Gain1 : Gain  Gain=360
  Gain2 : Gain  Gain=-1
  Rounding Function : Rounding
  Sum : Sum  Inputs=|-+
  Sum1 : Sum  Inputs=-+|
  Sum2 : Sum  Inputs=++|
  heading [deg] : Outport
  wires:
  Gain/1 -> Rounding Function/1
  Rounding Function/1 -> Gain1/1
  Gain1/1 -> Sum/1
  Angle [deg]/1 -> Sum1/2
  Sum/1 -> Gain2/1
  Sum1/1 -> Gain/1, Sum/2
  Constant/1 -> Sum1/1
  Gain2/1 -> Sum2/2
  Constant1/1 -> Sum2/1
  Sum2/1 -> heading [deg]/1

== system Demo_TrafficFlow_cs/HighwayOverhang_1/Throughput detection  Sensor/TrafficDensityCounter [cars/hour]/Subsystem ==
  Enable : EnablePort  PropagateVarSize=During execution
  Counter : Memory
  Increment Counter : Sum
  Ref_Range2 : Constant
  Out1 : Outport
  wires:
  Increment Counter/1 -> Out1/1, Counter/1
  Ref_Range2/1 -> Increment Counter/2
  Counter/1 -> Increment Counter/1

== system Demo_TrafficFlow_cs/HighwayOverhang_1/Throughput detection  Sensor/TrafficDensityCounter [cars/hour]/Subsystem1 ==
  Velocity [m/s] : Inport
  Enable : EnablePort  PropagateVarSize=During execution
  Gain : Gain  Gain=3.6
  Velocity [km/h] : Outport
  wires:
  Velocity [m/s]/1 -> Gain/1
  Gain/1 -> Velocity [km/h]/1

== system Demo_TrafficFlow_cs/HighwayOverhang_2/Antenna_Rx_3_Demux/compas converter/cart2compas ==
  Angle [deg] : Inport
  Constant : Constant  Value=90
  Constant1 : Constant  Value=360
  Gain : Gain  Gain=1/360
  Gain1 : Gain  Gain=360
  Gain2 : Gain  Gain=-1
  Rounding Function : Rounding
  Sum : Sum  Inputs=|-+
  Sum1 : Sum  Inputs=-+|
  Sum2 : Sum  Inputs=++|
  heading [deg] : Outport
  wires:
  Gain/1 -> Rounding Function/1
  Rounding Function/1 -> Gain1/1
  Gain1/1 -> Sum/1
  Angle [deg]/1 -> Sum1/2
  Sum/1 -> Gain2/1
  Sum1/1 -> Gain/1, Sum/2
  Constant/1 -> Sum1/1
  Gain2/1 -> Sum2/2
  Constant1/1 -> Sum2/1
  Sum2/1 -> heading [deg]/1

== system Demo_TrafficFlow_cs/HighwayOverhang_2/RFBeacon_2_Rx_Demux/compas converter/cart2compas ==
  Angle [deg] : Inport
  Constant : Constant  Value=90
  Constant1 : Constant  Value=360
  Gain : Gain  Gain=1/360
  Gain1 : Gain  Gain=360
  Gain2 : Gain  Gain=-1
  Rounding Function : Rounding
  Sum : Sum  Inputs=|-+
  Sum1 : Sum  Inputs=-+|
  Sum2 : Sum  Inputs=++|
  heading [deg] : Outport
  wires:
  Gain/1 -> Rounding Function/1
  Rounding Function/1 -> Gain1/1
  Gain1/1 -> Sum/1
  Angle [deg]/1 -> Sum1/2
  Sum/1 -> Gain2/1
  Sum1/1 -> Gain/1, Sum/2
  Constant/1 -> Sum1/1
  Gain2/1 -> Sum2/2
  Constant1/1 -> Sum2/1
  Sum2/1 -> heading [deg]/1

== system Demo_TrafficFlow_cs/HighwayOverhang_2/Throughput detection  Sensor/TrafficDensityCounter [cars/hour]/Subsystem ==
  Enable : EnablePort  PropagateVarSize=During execution
  Counter : Memory
  Increment Counter : Sum
  Ref_Range2 : Constant
  Out1 : Outport
  wires:
  Increment Counter/1 -> Out1/1, Counter/1
  Ref_Range2/1 -> Increment Counter/2
  Counter/1 -> Increment Counter/1

== system Demo_TrafficFlow_cs/HighwayOverhang_2/Throughput detection  Sensor/TrafficDensityCounter [cars/hour]/Subsystem1 ==
  Velocity [m/s] : Inport
  Enable : EnablePort  PropagateVarSize=During execution
  Gain : Gain  Gain=3.6
  Velocity [km/h] : Outport
  wires:
  Velocity [m/s]/1 -> Gain/1
  Gain/1 -> Velocity [km/h]/1

== system Demo_TrafficFlow_cs/HighwayOverhang_3/Antenna_Rx_4_Demux/compas converter/cart2compas ==
  Angle [deg] : Inport
  Constant : Constant  Value=90
  Constant1 : Constant  Value=360
  Gain : Gain  Gain=1/360
  Gain1 : Gain  Gain=360
  Gain2 : Gain  Gain=-1
  Rounding Function : Rounding
  Sum : Sum  Inputs=|-+
  Sum1 : Sum  Inputs=-+|
  Sum2 : Sum  Inputs=++|
  heading [deg] : Outport
  wires:
  Gain/1 -> Rounding Function/1
  Rounding Function/1 -> Gain1/1
  Gain1/1 -> Sum/1
  Angle [deg]/1 -> Sum1/2
  Sum/1 -> Gain2/1
  Sum1/1 -> Gain/1, Sum/2
  Constant/1 -> Sum1/1
  Gain2/1 -> Sum2/2
  Constant1/1 -> Sum2/1
  Sum2/1 -> heading [deg]/1

== system Demo_TrafficFlow_cs/HighwayOverhang_3/RFBeacon_3_Rx_Demux/compas converter/cart2compas ==
  Angle [deg] : Inport
  Constant : Constant  Value=90
  Constant1 : Constant  Value=360
  Gain : Gain  Gain=1/360
  Gain1 : Gain  Gain=360
  Gain2 : Gain  Gain=-1
  Rounding Function : Rounding
  Sum : Sum  Inputs=|-+
  Sum1 : Sum  Inputs=-+|
  Sum2 : Sum  Inputs=++|
  heading [deg] : Outport
  wires:
  Gain/1 -> Rounding Function/1
  Rounding Function/1 -> Gain1/1
  Gain1/1 -> Sum/1
  Angle [deg]/1 -> Sum1/2
  Sum/1 -> Gain2/1
  Sum1/1 -> Gain/1, Sum/2
  Constant/1 -> Sum1/1
  Gain2/1 -> Sum2/2
  Constant1/1 -> Sum2/1
  Sum2/1 -> heading [deg]/1

== system Demo_TrafficFlow_cs/HighwayOverhang_3/Throughput detection  Sensor/TrafficDensityCounter [cars/hour]/Subsystem ==
  Enable : EnablePort  PropagateVarSize=During execution
  Counter : Memory
  Increment Counter : Sum
  Ref_Range2 : Constant
  Out1 : Outport
  wires:
  Increment Counter/1 -> Out1/1, Counter/1
  Ref_Range2/1 -> Increment Counter/2
  Counter/1 -> Increment Counter/1

== system Demo_TrafficFlow_cs/HighwayOverhang_3/Throughput detection  Sensor/TrafficDensityCounter [cars/hour]/Subsystem1 ==
  Velocity [m/s] : Inport
  Enable : EnablePort  PropagateVarSize=During execution
  Gain : Gain  Gain=3.6
  Velocity [km/h] : Outport
  wires:
  Velocity [m/s]/1 -> Gain/1
  Gain/1 -> Velocity [km/h]/1

== system Demo_TrafficFlow_cs/Mazda_RX8_1/SELF_Demux/compas converter/cart2compas ==
  Angle [deg] : Inport
  Constant : Constant  Value=90
  Constant1 : Constant  Value=360
  Gain : Gain  Gain=1/360
  Gain1 : Gain  Gain=360
  Gain2 : Gain  Gain=-1
  Rounding Function : Rounding
  Sum : Sum  Inputs=|-+
  Sum1 : Sum  Inputs=-+|
  Sum2 : Sum  Inputs=++|
  heading [deg] : Outport
  wires:
  Sum2/1 -> heading [deg]/1
  Constant1/1 -> Sum2/1
  Gain2/1 -> Sum2/2
  Constant/1 -> Sum1/1
  Sum1/1 -> Sum/2, Gain/1
  Sum/1 -> Gain2/1
  Angle [deg]/1 -> Sum1/2
  Gain1/1 -> Sum/1
  Rounding Function/1 -> Gain1/1
  Gain/1 -> Rounding Function/1

== system Demo_TrafficFlow_cs/Mazda_RX8_1/Trajectories/ElapsedTrack1/MuxState ==
  xyzPos_[m] : Inport
  RPYAngle_[rad] : Inport  Port=2
  Demux : Demux  Outputs=3
  Discrete Derivative : simulink/Discrete/Discrete Derivative  SystemSampleTime=-1  gainval=1.0  ICPrevScaledInput=0.0  OutMin=[]  OutMax=[]  OutDataType=fixdt(1, 16)  OutScaling=2^-10  DoSatur=off
  Mux : Mux  Inputs=3
  Mux1 : Mux  Inputs=3
  Substitute 90 & 270 deg (and its plurals) : Subsystem
  State : Outport
  wires:
  Mux1/1 -> Mux/3
  Discrete Derivative/1 -> Mux/2
  RPYAngle_[rad]/1 -> Demux/1
  Demux/3 -> Mux1/3
  Demux/2 -> Substitute 90 & 270 deg (and its plurals)/1(Angle [rad])
  Demux/1 -> Mux1/1
  xyzPos_[m]/1 -> Discrete Derivative/1, Mux/1
  Mux/1 -> State/1
  Substitute 90 & 270 deg (and its plurals)/1(Subst Angle [rad]) -> Mux1/2

== system Demo_TrafficFlow_cs/Mazda_RX8_1/Trajectories/ElapsedTrack1/OffsetCor ==
  x pos : Inport
  y pos : Inport  Port=2
  z pos : Inport  Port=3
  R angle : Inport  Port=4
  P angle : Inport  Port=5
  Y angle : Inport  Port=6
  Offset : Inport  Port=7
  ApplyRotation : PreScanSimulinkLibrary/ApplyRotation  SystemSampleTime=-1
  Demux : Demux  Outputs=6
  Mux : Mux  Inputs=2
  Mux1 : Mux  Inputs=3
  Mux2 : Mux  Inputs=3
  Mux3 : Mux  Inputs=3
  Selector2 : Selector  InputPortWidth=6  IndexOptions=Index vector (dialog)  Indices=[4]  OutputSizes=1
  Selector3 : Selector  InputPortWidth=6  IndexOptions=Index vector (dialog)  Indices=[5]  OutputSizes=1
  Selector4 : Selector  InputPortWidth=6  IndexOptions=Index vector (dialog)  Indices=[6]  OutputSizes=1
  Sum : Sum  Inputs=|++
  x pos_cor : Outport
  y pos_cor : Outport  Port=2
  z pos_cor : Outport  Port=3
  R angle_cor : Outport  Port=4
  P angle_cor : Outport  Port=5
  Y angle_cor : Outport  Port=6
  wires:
  Mux3/1 -> ApplyRotation/1
  ApplyRotation/1 -> Mux/1
  Mux2/1 -> ApplyRotation/2
  Selector4/1 -> Mux2/3
  Selector3/1 -> Mux2/2
  Selector2/1 -> Mux2/1
  Mux1/1 -> Mux/2
  Y angle/1 -> Mux1/3
  P angle/1 -> Mux1/2
  R angle/1 -> Mux1/1
  Demux/1 -> x pos_cor/1
  Demux/2 -> y pos_cor/1
  Demux/3 -> z pos_cor/1
  Demux/4 -> R angle_cor/1
  Demux/5 -> P angle_cor/1
  Demux/6 -> Y angle_cor/1
  z pos/1 -> Mux3/3
  y pos/1 -> Mux3/2
  x pos/1 -> Mux3/1
  Offset/1 -> Selector4/1, Selector3/1, Selector2/1, Sum/2
  Mux/1 -> Sum/1
  Sum/1 -> Demux/1

== system Demo_TrafficFlow_cs/Mazda_RX8_1/Trajectories/LANE CHANGE LEFT (cummulative y position;  multiple shift to left are possible)/Cumulative Lane Change ==
  reset cum value : Inport
  end LC reached : Inport  Port=2
  single LC value : Inport  Port=3
  Logical Operator1 : Logic  Operator=NOT
  Memory1 : Memory
  Memory2 : Memory
  Sum : Sum  Inputs=|++
  Triggered Subsystem : Subsystem  TreatAsAtomicUnit=on
  cum LC value : Outport  InitialOutput=0
  wires:
  Sum/1 -> cum LC value/1  "value"
  end LC reached/1 -> Triggered Subsystem/Trigger
  Triggered Subsystem/1(Out1) -> Memory2/1, Sum/1  "hold_value"
  Memory1/1 -> Triggered Subsystem/1(In1)
  Memory2/1 -> Triggered Subsystem/2(In2)
  single LC value/1 -> Sum/2, Memory1/1
  reset cum value/1 -> Logical Operator1/1
  Logical Operator1/1 -> Triggered Subsystem/Enable

== system Demo_TrafficFlow_cs/Mazda_RX8_1/Trajectories/LANE CHANGE LEFT (cummulative y position;  multiple shift to left are possible)/Single LaneChange_Left ==
  on/off [-] : Inport
  velocity [m/s] : Inport  Port=2
  Enable : EnablePort  PropagateVarSize=During execution
  ElapsedTrack2 : Subsystem  mask(PreScan Demux Block:){Filename='Path_LC_Left.mat'}
      mask prompts: Filename "Name path-file"
      mask init:
      | %route=load(['trajectories/',Filename]);
      | route=load(Filename);
      | s=route.path(1,:);
      | x=route.path(2,:);
      | y=route.path(3,:);
      | yaw=route.path(4,:);
  Gain : Gain  Gain="[0 1 0   0 1 0   0 0 1]"
  Integrator2 : Integrator  ExternalReset=rising  UpperSaturationLimit=100  LowerSaturationLimit=-1e-3
  Logical Operator1 : Logic  Operator=NOT  AllPortsSameDT=off  OutDataTypeStr=boolean
  Product1 : Product  RndMeth=Zero
  Relational Operator : RelationalOperator  Operator=<  OutDataTypeStr=boolean
  Trajectory_4_Offset1 : Constant  Value=[0 0 0]
  ini_cond3 : Constant  Value=s(end)
  end LC reached : Outport  InitialOutput=1
  single LC value : Outport  Port=2  InitialOutput=0
  wires:
  on/off [-]/1 -> Integrator2/2  "trigger"
  Relational Operator/1 -> Product1/2, Logical Operator1/1  "end_NOT_reached"
  ini_cond3/1 -> Relational Operator/2
  Gain/1 -> Product1/1
  Integrator2/1 -> Relational Operator/1, ElapsedTrack2/2(s)
  Trajectory_4_Offset1/1 -> ElapsedTrack2/1(Offset)
  ElapsedTrack2/1(state) -> Gain/1
  Product1/1 -> single LC value/1
  velocity [m/s]/1 -> Integrator2/1
  Logical Operator1/1 -> end LC reached/1  "end_reached"

== system Demo_TrafficFlow_cs/Mazda_RX8_1/Trajectories/LANE CHANGE LEFT (cummulative y position;  multiple shift to left are possible)/Trigger ==
  Trigger : TriggerPort
  ini_cond10 : Constant
  Out1 : Outport  InitialOutput=[0]
  wires:
  ini_cond10/1 -> Out1/1

== system Demo_TrafficFlow_cs/Mazda_RX8_1/Trajectories/Traj2State1/Traj2State2 ==
  traj : Inport
  MuxState : Subsystem
  Traj2MuxState_Ext : Subsystem
  state : Outport
  wires:
  Traj2MuxState_Ext/9(Y [rad]) -> MuxState/9(Y [rad])
  Traj2MuxState_Ext/8(P [rad]) -> MuxState/8(P [rad])
  Traj2MuxState_Ext/7(R [rad]) -> MuxState/7(R [rad])
  Traj2MuxState_Ext/6(dz [m/s]) -> MuxState/6(dz [m/s])
  Traj2MuxState_Ext/5(dy [m/s]) -> MuxState/5(dy [m/s])
  Traj2MuxState_Ext/4(dx [m/s]) -> MuxState/4(dx [m/s])
  Traj2MuxState_Ext/3(z [m]) -> MuxState/3(z [m])
  Traj2MuxState_Ext/2(y [m]) -> MuxState/2(y [m])
  Traj2MuxState_Ext/1(x [m]) -> MuxState/1(x [m])
  MuxState/1(state) -> state/1
  traj/1 -> Traj2MuxState_Ext/1(Traj)

== system Demo_TrafficFlow_cs/Motorcyclist_1/SELF_Demux/compas converter/cart2compas ==
  Angle [deg] : Inport
  Constant : Constant  Value=90
  Constant1 : Constant  Value=360
  Gain : Gain  Gain=1/360
  Gain1 : Gain  Gain=360
  Gain2 : Gain  Gain=-1
  Rounding Function : Rounding
  Sum : Sum  Inputs=|-+
  Sum1 : Sum  Inputs=-+|
  Sum2 : Sum  Inputs=++|
  heading [deg] : Outport
  wires:
  Sum2/1 -> heading [deg]/1
  Constant1/1 -> Sum2/1
  Gain2/1 -> Sum2/2
  Constant/1 -> Sum1/1
  Sum1/1 -> Sum/2, Gain/1
  Sum/1 -> Gain2/1
  Angle [deg]/1 -> Sum1/2
  Gain1/1 -> Sum/1
  Rounding Function/1 -> Gain1/1
  Gain/1 -> Rounding Function/1
